simulink model "LCTC_vTD8_Test_TC0_ref6_2007a_TC"  (Simulink 7.0)
settings: Solver=ode4  StartTime=0.0  StopTime=110  FixedStep=0.01  RelTol=1e-3
InitFcn:
  | PedalInterp_para
values from neighbouring files (symbols this model uses):
  ped_min = 5    [PedalInterp_para.m]
  T_max_Brake = 4000    [PedalInterp_para.m]
  TI = 0    [PAS_callback.m]    (also in PSTI_callback.m = [block '/TI'])
undefined symbols (not defined in the file or in its folder): fzgbib, m_ab_100pro, m_ab_70pro, PedalInterpretation, v_bereich
libraries: simulink x320
(part 19 of 50)

== system LCTC_vTD8_Test_TC0_ref6_2007a_TC/TestData 1/SR-PI-02.2 Acceleration pedal interpretation/v = const & phi_Acc increases & T_des_Drive < 0/Generate increase 7/Generate  limited increase for partition1 variant2 1/Subsystem ==
  In1 : Inport  OutDataType=sfix(16)  OutScaling=2^0
  In2 : Inport  Port=2  OutDataType=sfix(16)  OutScaling=2^0
  In3 : Inport  Port=3  OutDataType=sfix(16)  OutScaling=2^0
  Subsystem1 : Subsystem
  Subsystem10 : Subsystem
  Subsystem11 : Subsystem
  Subsystem12 : Subsystem
  Subsystem13 : Subsystem
  Subsystem14 : Subsystem
  Subsystem15 : Subsystem
  Subsystem16 : Subsystem
  Subsystem17 : Subsystem
  Subsystem18 : Subsystem
  Subsystem19 : Subsystem
  Subsystem2 : Subsystem
  Subsystem20 : Subsystem
  Subsystem21 : Subsystem
  Subsystem22 : Subsystem
  Subsystem23 : Subsystem
  Subsystem24 : Subsystem
  Subsystem25 : Subsystem
  Subsystem26 : Subsystem
  Subsystem27 : Subsystem
  Subsystem28 : Subsystem
  Subsystem29 : Subsystem
  Subsystem3 : Subsystem
  Subsystem30 : Subsystem
  Subsystem31 : Subsystem
  Subsystem32 : Subsystem
  Subsystem33 : Subsystem
  Subsystem34 : Subsystem
  Subsystem35 : Subsystem
  Subsystem36 : Subsystem
  Subsystem37 : Subsystem
  Subsystem38 : Subsystem
  Subsystem39 : Subsystem
  Subsystem4 : Subsystem
  Subsystem40 : Subsystem
  Subsystem41 : Subsystem
  Subsystem42 : Subsystem
  Subsystem43 : Subsystem
  Subsystem44 : Subsystem
  Subsystem45 : Subsystem
  Subsystem46 : Subsystem
  Subsystem47 : Subsystem
  Subsystem48 : Subsystem
  Subsystem49 : Subsystem
  Subsystem5 : Subsystem
  Subsystem50 : Subsystem
  Subsystem51 : Subsystem
  Subsystem52 : Subsystem
  Subsystem53 : Subsystem
  Subsystem54 : Subsystem
  Subsystem55 : Subsystem
  Subsystem56 : Subsystem
  Subsystem57 : Subsystem
  Subsystem58 : Subsystem
  Subsystem59 : Subsystem
  Subsystem6 : Subsystem
  Subsystem60 : Subsystem
  Subsystem61 : Subsystem
  Subsystem62 : Subsystem
  Subsystem63 : Subsystem
  Subsystem64 : Subsystem
  Subsystem65 : Subsystem
  Subsystem66 : Subsystem
  Subsystem67 : Subsystem
  Subsystem68 : Subsystem
  Subsystem69 : Subsystem
  Subsystem7 : Subsystem
  Subsystem70 : Subsystem
  Subsystem8 : Subsystem
  Subsystem9 : Subsystem
  Out1 : Outport  OutDataType=sfix(16)  OutScaling=2^0
  wires:
  Subsystem70/1(Out1) -> Out1/1
  Subsystem68/1(Out1) -> Subsystem59/2(In2)
  Subsystem50/1(Out1) -> Subsystem51/2(In2)
  Subsystem69/1(Out1) -> Subsystem70/2(In2)
  Subsystem66/1(Out1) -> Subsystem69/2(In2)
  Subsystem65/1(Out1) -> Subsystem66/2(In2)
  Subsystem64/1(Out1) -> Subsystem65/2(In2)
  Subsystem63/1(Out1) -> Subsystem64/2(In2)
  Subsystem62/1(Out1) -> Subsystem63/2(In2)
  Subsystem61/1(Out1) -> Subsystem62/2(In2)
  Subsystem60/1(Out1) -> Subsystem61/2(In2)
  Subsystem59/1(Out1) -> Subsystem60/2(In2)
  Subsystem67/1(Out1) -> Subsystem68/2(In2)
  Subsystem58/1(Out1) -> Subsystem67/2(In2)
  Subsystem57/1(Out1) -> Subsystem58/2(In2)
  Subsystem56/1(Out1) -> Subsystem57/2(In2)
  Subsystem55/1(Out1) -> Subsystem56/2(In2)
  Subsystem54/1(Out1) -> Subsystem55/2(In2)
  Subsystem53/1(Out1) -> Subsystem54/2(In2)
  Subsystem52/1(Out1) -> Subsystem53/2(In2)
  Subsystem51/1(Out1) -> Subsystem52/2(In2)
  Subsystem48/1(Out1) -> Subsystem35/2(In2)
  Subsystem46/1(Out1) -> Subsystem27/2(In2)
  Subsystem44/1(Out1) -> Subsystem19/2(In2)
  Subsystem49/1(Out1) -> Subsystem50/2(In2)
  Subsystem42/1(Out1) -> Subsystem49/2(In2)
  Subsystem41/1(Out1) -> Subsystem42/2(In2)
  Subsystem40/1(Out1) -> Subsystem41/2(In2)
  Subsystem39/1(Out1) -> Subsystem40/2(In2)
  Subsystem38/1(Out1) -> Subsystem39/2(In2)
  Subsystem37/1(Out1) -> Subsystem38/2(In2)
  Subsystem36/1(Out1) -> Subsystem37/2(In2)
  Subsystem35/1(Out1) -> Subsystem36/2(In2)
  Subsystem47/1(Out1) -> Subsystem48/2(In2)
  Subsystem34/1(Out1) -> Subsystem47/2(In2)
  Subsystem33/1(Out1) -> Subsystem34/2(In2)
  Subsystem32/1(Out1) -> Subsystem33/2(In2)
  Subsystem31/1(Out1) -> Subsystem32/2(In2)
  Subsystem30/1(Out1) -> Subsystem31/2(In2)
  Subsystem29/1(Out1) -> Subsystem30/2(In2)
  Subsystem28/1(Out1) -> Subsystem29/2(In2)
  Subsystem27/1(Out1) -> Subsystem28/2(In2)
  Subsystem45/1(Out1) -> Subsystem46/2(In2)
  Subsystem26/1(Out1) -> Subsystem45/2(In2)
  Subsystem25/1(Out1) -> Subsystem26/2(In2)
  Subsystem23/1(Out1) -> Subsystem24/2(In2)
  Subsystem24/1(Out1) -> Subsystem25/2(In2)
  Subsystem22/1(Out1) -> Subsystem23/2(In2)
  Subsystem21/1(Out1) -> Subsystem22/2(In2)
  Subsystem20/1(Out1) -> Subsystem21/2(In2)
  Subsystem19/1(Out1) -> Subsystem20/2(In2)
  Subsystem43/1(Out1) -> Subsystem44/2(In2)
  Subsystem18/1(Out1) -> Subsystem43/2(In2)
  Subsystem10/1(Out1) -> Subsystem11/2(In2)
  Subsystem17/1(Out1) -> Subsystem18/2(In2)
  Subsystem16/1(Out1) -> Subsystem17/2(In2)
  Subsystem15/1(Out1) -> Subsystem16/2(In2)
  Subsystem13/1(Out1) -> Subsystem14/2(In2)
  Subsystem14/1(Out1) -> Subsystem15/2(In2)
  Subsystem12/1(Out1) -> Subsystem13/2(In2)
  Subsystem11/1(Out1) -> Subsystem12/2(In2)
  Subsystem9/1(Out1) -> Subsystem10/2(In2)
  Subsystem8/1(Out1) -> Subsystem9/2(In2)
  Subsystem7/1(Out1) -> Subsystem8/2(In2)
  Subsystem6/1(Out1) -> Subsystem7/2(In2)
  Subsystem5/1(Out1) -> Subsystem6/2(In2)
  Subsystem4/1(Out1) -> Subsystem5/2(In2)
  Subsystem3/1(Out1) -> Subsystem4/2(In2)
  Subsystem2/1(Out1) -> Subsystem3/2(In2)
  Subsystem1/1(Out1) -> Subsystem2/2(In2)
  In3/1 -> Subsystem70/3(In3), Subsystem69/3(In3), Subsystem66/3(In3), Subsystem65/3(In3), Subsystem64/3(In3), Subsystem63/3(In3), Subsystem62/3(In3), Subsystem61/3(In3), Subsystem60/3(In3), Subsystem59/3(In3), Subsystem68/3(In3), Subsystem67/3(In3), Subsystem58/3(In3), Subsystem57/3(In3), Subsystem56/3(In3), Subsystem55/3(In3), Subsystem54/3(In3), Subsystem53/3(In3), Subsystem52/3(In3), Subsystem51/3(In3), Subsystem50/3(In3), Subsystem49/3(In3), Subsystem42/3(In3), Subsystem41/3(In3), Subsystem40/3(In3), Subsystem39/3(In3), Subsystem38/3(In3), Subsystem37/3(In3), Subsystem36/3(In3), Subsystem35/3(In3), Subsystem48/3(In3), Subsystem47/3(In3), Subsystem34/3(In3), Subsystem33/3(In3), Subsystem32/3(In3), Subsystem31/3(In3), Subsystem30/3(In3), Subsystem29/3(In3), Subsystem28/3(In3), Subsystem27/3(In3), Subsystem46/3(In3), Subsystem45/3(In3), Subsystem26/3(In3), Subsystem25/3(In3), Subsystem24/3(In3), Subsystem23/3(In3), Subsystem22/3(In3), Subsystem21/3(In3), Subsystem20/3(In3), Subsystem19/3(In3), Subsystem11/3(In3), Subsystem44/3(In3), Subsystem43/3(In3), Subsystem18/3(In3), Subsystem17/3(In3), Subsystem16/3(In3), Subsystem15/3(In3), Subsystem14/3(In3), Subsystem13/3(In3), Subsystem12/3(In3), Subsystem1/3(In3), Subsystem10/3(In3), Subsystem9/3(In3), Subsystem8/3(In3), Subsystem7/3(In3), Subsystem6/3(In3), Subsystem5/3(In3), Subsystem4/3(In3), Subsystem3/3(In3), Subsystem2/3(In3)
  In2/1 -> Subsystem1/2(In2)
  In1/1 -> Subsystem44/1(In1), Subsystem43/1(In1), Subsystem18/1(In1), Subsystem17/1(In1), Subsystem16/1(In1), Subsystem15/1(In1), Subsystem14/1(In1), Subsystem13/1(In1), Subsystem12/1(In1), Subsystem11/1(In1), Subsystem46/1(In1), Subsystem45/1(In1), Subsystem26/1(In1), Subsystem25/1(In1), Subsystem24/1(In1), Subsystem23/1(In1), Subsystem22/1(In1), Subsystem21/1(In1), Subsystem20/1(In1), Subsystem19/1(In1), Subsystem70/1(In1), Subsystem69/1(In1), Subsystem66/1(In1), Subsystem65/1(In1), Subsystem64/1(In1), Subsystem63/1(In1), Subsystem62/1(In1), Subsystem61/1(In1), Subsystem60/1(In1), Subsystem59/1(In1), Subsystem68/1(In1), Subsystem67/1(In1), Subsystem58/1(In1), Subsystem57/1(In1), Subsystem56/1(In1), Subsystem55/1(In1), Subsystem54/1(In1), Subsystem53/1(In1), Subsystem52/1(In1), Subsystem51/1(In1), Subsystem50/1(In1), Subsystem49/1(In1), Subsystem42/1(In1), Subsystem41/1(In1), Subsystem40/1(In1), Subsystem39/1(In1), Subsystem38/1(In1), Subsystem37/1(In1), Subsystem36/1(In1), Subsystem35/1(In1), Subsystem48/1(In1), Subsystem47/1(In1), Subsystem34/1(In1), Subsystem33/1(In1), Subsystem32/1(In1), Subsystem31/1(In1), Subsystem30/1(In1), Subsystem29/1(In1), Subsystem28/1(In1), Subsystem27/1(In1), Subsystem10/1(In1), Subsystem9/1(In1), Subsystem8/1(In1), Subsystem7/1(In1), Subsystem6/1(In1), Subsystem5/1(In1), Subsystem4/1(In1), Subsystem3/1(In1), Subsystem2/1(In1), Subsystem1/1(In1)

== system LCTC_vTD8_Test_TC0_ref6_2007a_TC/TestData 1/SR-PI-02.2 Acceleration pedal interpretation/v = const & phi_Acc increases & T_des_Drive >= 0/Generate constant 6/Generate  constant for partition1 variant1 1/Repeating Sequence ==
  Clock : Clock
      settings: Decimation=10
  Fcn1 : Fcn  Expr=rem(u[1],period)
  Look-Up Table1 : Lookup  InputValues=rep_seq_t - min(rep_seq_t)  Table=rep_seq_y  OutDataType=sfix(16)  OutScaling=2^0  SaturateOnIntegerOverflow=on
  Output : SignalConversion  ConversionOutput=Contiguous copy
  Sum : Sum  Inputs=+-  OutDataType=sfix(16)  OutScaling=2^0  SaturateOnIntegerOverflow=on  InputSameDT=on
  startTime : S-Function  FunctionName=sfun_tstart
  Out1 : Outport  OutDataType=sfix(16)  OutScaling=2^0
  wires:
  Output/1 -> Out1/1
  Look-Up Table1/1 -> Output/1
  startTime/1 -> Sum/2
  Clock/1 -> Sum/1
  Sum/1 -> Fcn1/1
  Fcn1/1 -> Look-Up Table1/1

== system LCTC_vTD8_Test_TC0_ref6_2007a_TC/TestData 1/SR-PI-02.2 Acceleration pedal interpretation/v = const & phi_Acc increases & T_des_Drive >= 0/Generate constant 6/Generate  constant for partition1 variant2 1/Repeating Sequence ==
  Clock : Clock
      settings: Decimation=10
  Fcn1 : Fcn  Expr=rem(u[1],period)
  Look-Up Table1 : Lookup  InputValues=rep_seq_t - min(rep_seq_t)  Table=rep_seq_y  OutDataType=sfix(16)  OutScaling=2^0  SaturateOnIntegerOverflow=on
  Output : SignalConversion  ConversionOutput=Contiguous copy
  Sum : Sum  Inputs=+-  OutDataType=sfix(16)  OutScaling=2^0  SaturateOnIntegerOverflow=on  InputSameDT=on
  startTime : S-Function  FunctionName=sfun_tstart
  Out1 : Outport  OutDataType=sfix(16)  OutScaling=2^0
  wires:
  Output/1 -> Out1/1
  Look-Up Table1/1 -> Output/1
  startTime/1 -> Sum/2
  Clock/1 -> Sum/1
  Sum/1 -> Fcn1/1
  Fcn1/1 -> Look-Up Table1/1

== system LCTC_vTD8_Test_TC0_ref6_2007a_TC/TestData 1/SR-PI-02.2 Acceleration pedal interpretation/v = const & phi_Acc increases & T_des_Drive >= 0/Generate constant 6/Generate  constant for partition1 variant3 1/Repeating Sequence ==
  Clock : Clock
      settings: Decimation=10
  Fcn1 : Fcn  Expr=rem(u[1],period)
  Look-Up Table1 : Lookup  InputValues=rep_seq_t - min(rep_seq_t)  Table=rep_seq_y  OutDataType=sfix(16)  OutScaling=2^0  SaturateOnIntegerOverflow=on
  Output : SignalConversion  ConversionOutput=Contiguous copy
  Sum : Sum  Inputs=+-  OutDataType=sfix(16)  OutScaling=2^0  SaturateOnIntegerOverflow=on  InputSameDT=on
  startTime : S-Function  FunctionName=sfun_tstart
  Out1 : Outport  OutDataType=sfix(16)  OutScaling=2^0
  wires:
  Output/1 -> Out1/1
  Look-Up Table1/1 -> Output/1
  startTime/1 -> Sum/2
  Clock/1 -> Sum/1
  Sum/1 -> Fcn1/1
  Fcn1/1 -> Look-Up Table1/1

== system LCTC_vTD8_Test_TC0_ref6_2007a_TC/TestData 1/SR-PI-02.2 Acceleration pedal interpretation/v = const & phi_Acc increases & T_des_Drive >= 0/Generate constant 6/Generate  constant for partition1 variant4 1/Repeating Sequence ==
  Clock : Clock
      settings: Decimation=10
  Fcn1 : Fcn  Expr=rem(u[1],period)
  Look-Up Table1 : Lookup  InputValues=rep_seq_t - min(rep_seq_t)  Table=rep_seq_y  OutDataType=sfix(16)  OutScaling=2^0  SaturateOnIntegerOverflow=on
  Output : SignalConversion  ConversionOutput=Contiguous copy
  Sum : Sum  Inputs=+-  OutDataType=sfix(16)  OutScaling=2^0  SaturateOnIntegerOverflow=on  InputSameDT=on
  startTime : S-Function  FunctionName=sfun_tstart
  Out1 : Outport  OutDataType=sfix(16)  OutScaling=2^0
  wires:
  Output/1 -> Out1/1
  Look-Up Table1/1 -> Output/1
  startTime/1 -> Sum/2
  Clock/1 -> Sum/1
  Sum/1 -> Fcn1/1
  Fcn1/1 -> Look-Up Table1/1

== system LCTC_vTD8_Test_TC0_ref6_2007a_TC/TestData 1/SR-PI-02.2 Acceleration pedal interpretation/v = const & phi_Acc increases & T_des_Drive >= 0/Generate constant 6/Generate  constant for partition1 variant5 1/Repeating Sequence ==
  Clock : Clock
      settings: Decimation=10
  Fcn1 : Fcn  Expr=rem(u[1],period)
  Look-Up Table1 : Lookup  InputValues=rep_seq_t - min(rep_seq_t)  Table=rep_seq_y  OutDataType=sfix(16)  OutScaling=2^0  SaturateOnIntegerOverflow=on
  Output : SignalConversion  ConversionOutput=Contiguous copy
  Sum : Sum  Inputs=+-  OutDataType=sfix(16)  OutScaling=2^0  SaturateOnIntegerOverflow=on  InputSameDT=on
  startTime : S-Function  FunctionName=sfun_tstart
  Out1 : Outport  OutDataType=sfix(16)  OutScaling=2^0
  wires:
  Output/1 -> Out1/1
  Look-Up Table1/1 -> Output/1
  startTime/1 -> Sum/2
  Clock/1 -> Sum/1
  Sum/1 -> Fcn1/1
  Fcn1/1 -> Look-Up Table1/1

== system LCTC_vTD8_Test_TC0_ref6_2007a_TC/TestData 1/SR-PI-02.2 Acceleration pedal interpretation/v = const & phi_Acc increases & T_des_Drive >= 0/Generate increase 7/Generate  limited increase for partition1 variant1 1/Subsystem ==
  In1 : Inport  OutDataType=sfix(16)  OutScaling=2^0
  In2 : Inport  Port=2  OutDataType=sfix(16)  OutScaling=2^0
  In3 : Inport  Port=3  OutDataType=sfix(16)  OutScaling=2^0
  Subsystem1 : Subsystem
  Subsystem10 : Subsystem
  Subsystem11 : Subsystem
  Subsystem12 : Subsystem
  Subsystem13 : Subsystem
  Subsystem14 : Subsystem
  Subsystem15 : Subsystem
  Subsystem16 : Subsystem
  Subsystem17 : Subsystem
  Subsystem18 : Subsystem
  Subsystem19 : Subsystem
  Subsystem2 : Subsystem
  Subsystem20 : Subsystem
  Subsystem21 : Subsystem
  Subsystem22 : Subsystem
  Subsystem23 : Subsystem
  Subsystem24 : Subsystem
  Subsystem25 : Subsystem
  Subsystem26 : Subsystem
  Subsystem27 : Subsystem
  Subsystem28 : Subsystem
  Subsystem29 : Subsystem
  Subsystem3 : Subsystem
  Subsystem30 : Subsystem
  Subsystem31 : Subsystem
  Subsystem32 : Subsystem
  Subsystem33 : Subsystem
  Subsystem34 : Subsystem
  Subsystem35 : Subsystem
  Subsystem36 : Subsystem
  Subsystem37 : Subsystem
  Subsystem38 : Subsystem
  Subsystem39 : Subsystem
  Subsystem4 : Subsystem
  Subsystem40 : Subsystem
  Subsystem41 : Subsystem
  Subsystem42 : Subsystem
  Subsystem43 : Subsystem
  Subsystem44 : Subsystem
  Subsystem45 : Subsystem
  Subsystem46 : Subsystem
  Subsystem47 : Subsystem
  Subsystem48 : Subsystem
  Subsystem49 : Subsystem
  Subsystem5 : Subsystem
  Subsystem50 : Subsystem
  Subsystem51 : Subsystem
  Subsystem52 : Subsystem
  Subsystem53 : Subsystem
  Subsystem54 : Subsystem
  Subsystem55 : Subsystem
  Subsystem56 : Subsystem
  Subsystem57 : Subsystem
  Subsystem58 : Subsystem
  Subsystem59 : Subsystem
  Subsystem6 : Subsystem
  Subsystem60 : Subsystem
  Subsystem61 : Subsystem
  Subsystem62 : Subsystem
  Subsystem63 : Subsystem
  Subsystem64 : Subsystem
  Subsystem65 : Subsystem
  Subsystem66 : Subsystem
  Subsystem67 : Subsystem
  Subsystem68 : Subsystem
  Subsystem69 : Subsystem
  Subsystem7 : Subsystem
  Subsystem70 : Subsystem
  Subsystem8 : Subsystem
  Subsystem9 : Subsystem
  Out1 : Outport  OutDataType=sfix(16)  OutScaling=2^0
  wires:
  Subsystem70/1(Out1) -> Out1/1
  Subsystem68/1(Out1) -> Subsystem59/2(In2)
  Subsystem50/1(Out1) -> Subsystem51/2(In2)
  Subsystem69/1(Out1) -> Subsystem70/2(In2)
  Subsystem66/1(Out1) -> Subsystem69/2(In2)
  Subsystem65/1(Out1) -> Subsystem66/2(In2)
  Subsystem64/1(Out1) -> Subsystem65/2(In2)
  Subsystem63/1(Out1) -> Subsystem64/2(In2)
  Subsystem62/1(Out1) -> Subsystem63/2(In2)
  Subsystem61/1(Out1) -> Subsystem62/2(In2)
  Subsystem60/1(Out1) -> Subsystem61/2(In2)
  Subsystem59/1(Out1) -> Subsystem60/2(In2)
  Subsystem67/1(Out1) -> Subsystem68/2(In2)
  Subsystem58/1(Out1) -> Subsystem67/2(In2)
  Subsystem57/1(Out1) -> Subsystem58/2(In2)
  Subsystem56/1(Out1) -> Subsystem57/2(In2)
  Subsystem55/1(Out1) -> Subsystem56/2(In2)
  Subsystem54/1(Out1) -> Subsystem55/2(In2)
  Subsystem53/1(Out1) -> Subsystem54/2(In2)
  Subsystem52/1(Out1) -> Subsystem53/2(In2)
  Subsystem51/1(Out1) -> Subsystem52/2(In2)
  Subsystem48/1(Out1) -> Subsystem35/2(In2)
  Subsystem46/1(Out1) -> Subsystem27/2(In2)
  Subsystem44/1(Out1) -> Subsystem19/2(In2)
  Subsystem49/1(Out1) -> Subsystem50/2(In2)
  Subsystem42/1(Out1) -> Subsystem49/2(In2)
  Subsystem41/1(Out1) -> Subsystem42/2(In2)
  Subsystem40/1(Out1) -> Subsystem41/2(In2)
  Subsystem39/1(Out1) -> Subsystem40/2(In2)
  Subsystem38/1(Out1) -> Subsystem39/2(In2)
  Subsystem37/1(Out1) -> Subsystem38/2(In2)
  Subsystem36/1(Out1) -> Subsystem37/2(In2)
  Subsystem35/1(Out1) -> Subsystem36/2(In2)
  Subsystem47/1(Out1) -> Subsystem48/2(In2)
  Subsystem34/1(Out1) -> Subsystem47/2(In2)
  Subsystem33/1(Out1) -> Subsystem34/2(In2)
  Subsystem32/1(Out1) -> Subsystem33/2(In2)
  Subsystem31/1(Out1) -> Subsystem32/2(In2)
  Subsystem30/1(Out1) -> Subsystem31/2(In2)
  Subsystem29/1(Out1) -> Subsystem30/2(In2)
  Subsystem28/1(Out1) -> Subsystem29/2(In2)
  Subsystem27/1(Out1) -> Subsystem28/2(In2)
  Subsystem45/1(Out1) -> Subsystem46/2(In2)
  Subsystem26/1(Out1) -> Subsystem45/2(In2)
  Subsystem25/1(Out1) -> Subsystem26/2(In2)
  Subsystem23/1(Out1) -> Subsystem24/2(In2)
  Subsystem24/1(Out1) -> Subsystem25/2(In2)
  Subsystem22/1(Out1) -> Subsystem23/2(In2)
  Subsystem21/1(Out1) -> Subsystem22/2(In2)
  Subsystem20/1(Out1) -> Subsystem21/2(In2)
  Subsystem19/1(Out1) -> Subsystem20/2(In2)
  Subsystem43/1(Out1) -> Subsystem44/2(In2)
  Subsystem18/1(Out1) -> Subsystem43/2(In2)
  Subsystem10/1(Out1) -> Subsystem11/2(In2)
  Subsystem17/1(Out1) -> Subsystem18/2(In2)
  Subsystem16/1(Out1) -> Subsystem17/2(In2)
  Subsystem15/1(Out1) -> Subsystem16/2(In2)
  Subsystem13/1(Out1) -> Subsystem14/2(In2)
  Subsystem14/1(Out1) -> Subsystem15/2(In2)
  Subsystem12/1(Out1) -> Subsystem13/2(In2)
  Subsystem11/1(Out1) -> Subsystem12/2(In2)
  Subsystem9/1(Out1) -> Subsystem10/2(In2)
  Subsystem8/1(Out1) -> Subsystem9/2(In2)
  Subsystem7/1(Out1) -> Subsystem8/2(In2)
  Subsystem6/1(Out1) -> Subsystem7/2(In2)
  Subsystem5/1(Out1) -> Subsystem6/2(In2)
  Subsystem4/1(Out1) -> Subsystem5/2(In2)
  Subsystem3/1(Out1) -> Subsystem4/2(In2)
  Subsystem2/1(Out1) -> Subsystem3/2(In2)
  Subsystem1/1(Out1) -> Subsystem2/2(In2)
  In3/1 -> Subsystem70/3(In3), Subsystem69/3(In3), Subsystem66/3(In3), Subsystem65/3(In3), Subsystem64/3(In3), Subsystem63/3(In3), Subsystem62/3(In3), Subsystem61/3(In3), Subsystem60/3(In3), Subsystem59/3(In3), Subsystem68/3(In3), Subsystem67/3(In3), Subsystem58/3(In3), Subsystem57/3(In3), Subsystem56/3(In3), Subsystem55/3(In3), Subsystem54/3(In3), Subsystem53/3(In3), Subsystem52/3(In3), Subsystem51/3(In3), Subsystem50/3(In3), Subsystem49/3(In3), Subsystem42/3(In3), Subsystem41/3(In3), Subsystem40/3(In3), Subsystem39/3(In3), Subsystem38/3(In3), Subsystem37/3(In3), Subsystem36/3(In3), Subsystem35/3(In3), Subsystem48/3(In3), Subsystem47/3(In3), Subsystem34/3(In3), Subsystem33/3(In3), Subsystem32/3(In3), Subsystem31/3(In3), Subsystem30/3(In3), Subsystem29/3(In3), Subsystem28/3(In3), Subsystem27/3(In3), Subsystem46/3(In3), Subsystem45/3(In3), Subsystem26/3(In3), Subsystem25/3(In3), Subsystem24/3(In3), Subsystem23/3(In3), Subsystem22/3(In3), Subsystem21/3(In3), Subsystem20/3(In3), Subsystem19/3(In3), Subsystem11/3(In3), Subsystem44/3(In3), Subsystem43/3(In3), Subsystem18/3(In3), Subsystem17/3(In3), Subsystem16/3(In3), Subsystem15/3(In3), Subsystem14/3(In3), Subsystem13/3(In3), Subsystem12/3(In3), Subsystem1/3(In3), Subsystem10/3(In3), Subsystem9/3(In3), Subsystem8/3(In3), Subsystem7/3(In3), Subsystem6/3(In3), Subsystem5/3(In3), Subsystem4/3(In3), Subsystem3/3(In3), Subsystem2/3(In3)
  In2/1 -> Subsystem1/2(In2)
  In1/1 -> Subsystem44/1(In1), Subsystem43/1(In1), Subsystem18/1(In1), Subsystem17/1(In1), Subsystem16/1(In1), Subsystem15/1(In1), Subsystem14/1(In1), Subsystem13/1(In1), Subsystem12/1(In1), Subsystem11/1(In1), Subsystem46/1(In1), Subsystem45/1(In1), Subsystem26/1(In1), Subsystem25/1(In1), Subsystem24/1(In1), Subsystem23/1(In1), Subsystem22/1(In1), Subsystem21/1(In1), Subsystem20/1(In1), Subsystem19/1(In1), Subsystem70/1(In1), Subsystem69/1(In1), Subsystem66/1(In1), Subsystem65/1(In1), Subsystem64/1(In1), Subsystem63/1(In1), Subsystem62/1(In1), Subsystem61/1(In1), Subsystem60/1(In1), Subsystem59/1(In1), Subsystem68/1(In1), Subsystem67/1(In1), Subsystem58/1(In1), Subsystem57/1(In1), Subsystem56/1(In1), Subsystem55/1(In1), Subsystem54/1(In1), Subsystem53/1(In1), Subsystem52/1(In1), Subsystem51/1(In1), Subsystem50/1(In1), Subsystem49/1(In1), Subsystem42/1(In1), Subsystem41/1(In1), Subsystem40/1(In1), Subsystem39/1(In1), Subsystem38/1(In1), Subsystem37/1(In1), Subsystem36/1(In1), Subsystem35/1(In1), Subsystem48/1(In1), Subsystem47/1(In1), Subsystem34/1(In1), Subsystem33/1(In1), Subsystem32/1(In1), Subsystem31/1(In1), Subsystem30/1(In1), Subsystem29/1(In1), Subsystem28/1(In1), Subsystem27/1(In1), Subsystem10/1(In1), Subsystem9/1(In1), Subsystem8/1(In1), Subsystem7/1(In1), Subsystem6/1(In1), Subsystem5/1(In1), Subsystem4/1(In1), Subsystem3/1(In1), Subsystem2/1(In1), Subsystem1/1(In1)

== system LCTC_vTD8_Test_TC0_ref6_2007a_TC/TestData 1/SR-PI-02.2 Acceleration pedal interpretation/v = const & phi_Acc increases & T_des_Drive >= 0/Generate increase 7/Generate  limited increase for partition1 variant2 1/Subsystem ==
  In1 : Inport  OutDataType=sfix(16)  OutScaling=2^0
  In2 : Inport  Port=2  OutDataType=sfix(16)  OutScaling=2^0
  In3 : Inport  Port=3  OutDataType=sfix(16)  OutScaling=2^0
  Subsystem1 : Subsystem
  Subsystem10 : Subsystem
  Subsystem11 : Subsystem
  Subsystem12 : Subsystem
  Subsystem13 : Subsystem
  Subsystem14 : Subsystem
  Subsystem15 : Subsystem
  Subsystem16 : Subsystem
  Subsystem17 : Subsystem
  Subsystem18 : Subsystem
  Subsystem19 : Subsystem
  Subsystem2 : Subsystem
  Subsystem20 : Subsystem
  Subsystem21 : Subsystem
  Subsystem22 : Subsystem
  Subsystem23 : Subsystem
  Subsystem24 : Subsystem
  Subsystem25 : Subsystem
  Subsystem26 : Subsystem
  Subsystem27 : Subsystem
  Subsystem28 : Subsystem
  Subsystem29 : Subsystem
  Subsystem3 : Subsystem
  Subsystem30 : Subsystem
  Subsystem31 : Subsystem
  Subsystem32 : Subsystem
  Subsystem33 : Subsystem
  Subsystem34 : Subsystem
  Subsystem35 : Subsystem
  Subsystem36 : Subsystem
  Subsystem37 : Subsystem
  Subsystem38 : Subsystem
  Subsystem39 : Subsystem
  Subsystem4 : Subsystem
  Subsystem40 : Subsystem
  Subsystem41 : Subsystem
  Subsystem42 : Subsystem
  Subsystem43 : Subsystem
  Subsystem44 : Subsystem
  Subsystem45 : Subsystem
  Subsystem46 : Subsystem
  Subsystem47 : Subsystem
  Subsystem48 : Subsystem
  Subsystem49 : Subsystem
  Subsystem5 : Subsystem
  Subsystem50 : Subsystem
  Subsystem51 : Subsystem
  Subsystem52 : Subsystem
  Subsystem53 : Subsystem
  Subsystem54 : Subsystem
  Subsystem55 : Subsystem
  Subsystem56 : Subsystem
  Subsystem57 : Subsystem
  Subsystem58 : Subsystem
  Subsystem59 : Subsystem
  Subsystem6 : Subsystem
  Subsystem60 : Subsystem
  Subsystem61 : Subsystem
  Subsystem62 : Subsystem
  Subsystem63 : Subsystem
  Subsystem64 : Subsystem
  Subsystem65 : Subsystem
  Subsystem66 : Subsystem
  Subsystem67 : Subsystem
  Subsystem68 : Subsystem
  Subsystem69 : Subsystem
  Subsystem7 : Subsystem
  Subsystem70 : Subsystem
  Subsystem8 : Subsystem
  Subsystem9 : Subsystem
  Out1 : Outport  OutDataType=sfix(16)  OutScaling=2^0
  wires:
  Subsystem70/1(Out1) -> Out1/1
  Subsystem68/1(Out1) -> Subsystem59/2(In2)
  Subsystem50/1(Out1) -> Subsystem51/2(In2)
  Subsystem69/1(Out1) -> Subsystem70/2(In2)
  Subsystem66/1(Out1) -> Subsystem69/2(In2)
  Subsystem65/1(Out1) -> Subsystem66/2(In2)
  Subsystem64/1(Out1) -> Subsystem65/2(In2)
  Subsystem63/1(Out1) -> Subsystem64/2(In2)
  Subsystem62/1(Out1) -> Subsystem63/2(In2)
  Subsystem61/1(Out1) -> Subsystem62/2(In2)
  Subsystem60/1(Out1) -> Subsystem61/2(In2)
  Subsystem59/1(Out1) -> Subsystem60/2(In2)
  Subsystem67/1(Out1) -> Subsystem68/2(In2)
  Subsystem58/1(Out1) -> Subsystem67/2(In2)
  Subsystem57/1(Out1) -> Subsystem58/2(In2)
  Subsystem56/1(Out1) -> Subsystem57/2(In2)
  Subsystem55/1(Out1) -> Subsystem56/2(In2)
  Subsystem54/1(Out1) -> Subsystem55/2(In2)
  Subsystem53/1(Out1) -> Subsystem54/2(In2)
  Subsystem52/1(Out1) -> Subsystem53/2(In2)
  Subsystem51/1(Out1) -> Subsystem52/2(In2)
  Subsystem48/1(Out1) -> Subsystem35/2(In2)
  Subsystem46/1(Out1) -> Subsystem27/2(In2)
  Subsystem44/1(Out1) -> Subsystem19/2(In2)
  Subsystem49/1(Out1) -> Subsystem50/2(In2)
  Subsystem42/1(Out1) -> Subsystem49/2(In2)
  Subsystem41/1(Out1) -> Subsystem42/2(In2)
  Subsystem40/1(Out1) -> Subsystem41/2(In2)
  Subsystem39/1(Out1) -> Subsystem40/2(In2)
  Subsystem38/1(Out1) -> Subsystem39/2(In2)
  Subsystem37/1(Out1) -> Subsystem38/2(In2)
  Subsystem36/1(Out1) -> Subsystem37/2(In2)
  Subsystem35/1(Out1) -> Subsystem36/2(In2)
  Subsystem47/1(Out1) -> Subsystem48/2(In2)
  Subsystem34/1(Out1) -> Subsystem47/2(In2)
  Subsystem33/1(Out1) -> Subsystem34/2(In2)
  Subsystem32/1(Out1) -> Subsystem33/2(In2)
  Subsystem31/1(Out1) -> Subsystem32/2(In2)
  Subsystem30/1(Out1) -> Subsystem31/2(In2)
  Subsystem29/1(Out1) -> Subsystem30/2(In2)
  Subsystem28/1(Out1) -> Subsystem29/2(In2)
  Subsystem27/1(Out1) -> Subsystem28/2(In2)
  Subsystem45/1(Out1) -> Subsystem46/2(In2)
  Subsystem26/1(Out1) -> Subsystem45/2(In2)
  Subsystem25/1(Out1) -> Subsystem26/2(In2)
  Subsystem23/1(Out1) -> Subsystem24/2(In2)
  Subsystem24/1(Out1) -> Subsystem25/2(In2)
  Subsystem22/1(Out1) -> Subsystem23/2(In2)
  Subsystem21/1(Out1) -> Subsystem22/2(In2)
  Subsystem20/1(Out1) -> Subsystem21/2(In2)
  Subsystem19/1(Out1) -> Subsystem20/2(In2)
  Subsystem43/1(Out1) -> Subsystem44/2(In2)
  Subsystem18/1(Out1) -> Subsystem43/2(In2)
  Subsystem10/1(Out1) -> Subsystem11/2(In2)
  Subsystem17/1(Out1) -> Subsystem18/2(In2)
  Subsystem16/1(Out1) -> Subsystem17/2(In2)
  Subsystem15/1(Out1) -> Subsystem16/2(In2)
  Subsystem13/1(Out1) -> Subsystem14/2(In2)
  Subsystem14/1(Out1) -> Subsystem15/2(In2)
  Subsystem12/1(Out1) -> Subsystem13/2(In2)
  Subsystem11/1(Out1) -> Subsystem12/2(In2)
  Subsystem9/1(Out1) -> Subsystem10/2(In2)
  Subsystem8/1(Out1) -> Subsystem9/2(In2)
  Subsystem7/1(Out1) -> Subsystem8/2(In2)
  Subsystem6/1(Out1) -> Subsystem7/2(In2)
  Subsystem5/1(Out1) -> Subsystem6/2(In2)
  Subsystem4/1(Out1) -> Subsystem5/2(In2)
  Subsystem3/1(Out1) -> Subsystem4/2(In2)
  Subsystem2/1(Out1) -> Subsystem3/2(In2)
  Subsystem1/1(Out1) -> Subsystem2/2(In2)
  In3/1 -> Subsystem70/3(In3), Subsystem69/3(In3), Subsystem66/3(In3), Subsystem65/3(In3), Subsystem64/3(In3), Subsystem63/3(In3), Subsystem62/3(In3), Subsystem61/3(In3), Subsystem60/3(In3), Subsystem59/3(In3), Subsystem68/3(In3), Subsystem67/3(In3), Subsystem58/3(In3), Subsystem57/3(In3), Subsystem56/3(In3), Subsystem55/3(In3), Subsystem54/3(In3), Subsystem53/3(In3), Subsystem52/3(In3), Subsystem51/3(In3), Subsystem50/3(In3), Subsystem49/3(In3), Subsystem42/3(In3), Subsystem41/3(In3), Subsystem40/3(In3), Subsystem39/3(In3), Subsystem38/3(In3), Subsystem37/3(In3), Subsystem36/3(In3), Subsystem35/3(In3), Subsystem48/3(In3), Subsystem47/3(In3), Subsystem34/3(In3), Subsystem33/3(In3), Subsystem32/3(In3), Subsystem31/3(In3), Subsystem30/3(In3), Subsystem29/3(In3), Subsystem28/3(In3), Subsystem27/3(In3), Subsystem46/3(In3), Subsystem45/3(In3), Subsystem26/3(In3), Subsystem25/3(In3), Subsystem24/3(In3), Subsystem23/3(In3), Subsystem22/3(In3), Subsystem21/3(In3), Subsystem20/3(In3), Subsystem19/3(In3), Subsystem11/3(In3), Subsystem44/3(In3), Subsystem43/3(In3), Subsystem18/3(In3), Subsystem17/3(In3), Subsystem16/3(In3), Subsystem15/3(In3), Subsystem14/3(In3), Subsystem13/3(In3), Subsystem12/3(In3), Subsystem1/3(In3), Subsystem10/3(In3), Subsystem9/3(In3), Subsystem8/3(In3), Subsystem7/3(In3), Subsystem6/3(In3), Subsystem5/3(In3), Subsystem4/3(In3), Subsystem3/3(In3), Subsystem2/3(In3)
  In2/1 -> Subsystem1/2(In2)
  In1/1 -> Subsystem44/1(In1), Subsystem43/1(In1), Subsystem18/1(In1), Subsystem17/1(In1), Subsystem16/1(In1), Subsystem15/1(In1), Subsystem14/1(In1), Subsystem13/1(In1), Subsystem12/1(In1), Subsystem11/1(In1), Subsystem46/1(In1), Subsystem45/1(In1), Subsystem26/1(In1), Subsystem25/1(In1), Subsystem24/1(In1), Subsystem23/1(In1), Subsystem22/1(In1), Subsystem21/1(In1), Subsystem20/1(In1), Subsystem19/1(In1), Subsystem70/1(In1), Subsystem69/1(In1), Subsystem66/1(In1), Subsystem65/1(In1), Subsystem64/1(In1), Subsystem63/1(In1), Subsystem62/1(In1), Subsystem61/1(In1), Subsystem60/1(In1), Subsystem59/1(In1), Subsystem68/1(In1), Subsystem67/1(In1), Subsystem58/1(In1), Subsystem57/1(In1), Subsystem56/1(In1), Subsystem55/1(In1), Subsystem54/1(In1), Subsystem53/1(In1), Subsystem52/1(In1), Subsystem51/1(In1), Subsystem50/1(In1), Subsystem49/1(In1), Subsystem42/1(In1), Subsystem41/1(In1), Subsystem40/1(In1), Subsystem39/1(In1), Subsystem38/1(In1), Subsystem37/1(In1), Subsystem36/1(In1), Subsystem35/1(In1), Subsystem48/1(In1), Subsystem47/1(In1), Subsystem34/1(In1), Subsystem33/1(In1), Subsystem32/1(In1), Subsystem31/1(In1), Subsystem30/1(In1), Subsystem29/1(In1), Subsystem28/1(In1), Subsystem27/1(In1), Subsystem10/1(In1), Subsystem9/1(In1), Subsystem8/1(In1), Subsystem7/1(In1), Subsystem6/1(In1), Subsystem5/1(In1), Subsystem4/1(In1), Subsystem3/1(In1), Subsystem2/1(In1), Subsystem1/1(In1)

== system LCTC_vTD8_Test_TC0_ref6_2007a_TC/TestData 1/SR-PI-02.2 Acceleration pedal interpretation/v increases & phi_Acc = const & T_des_Drive >= 0/Generate constant 6/Generate  constant for partition1 variant1 1/Repeating Sequence ==
  Clock : Clock
      settings: Decimation=10
  Fcn1 : Fcn  Expr=rem(u[1],period)
  Look-Up Table1 : Lookup  InputValues=rep_seq_t - min(rep_seq_t)  Table=rep_seq_y  OutDataType=sfix(16)  OutScaling=2^0  SaturateOnIntegerOverflow=on
  Output : SignalConversion  ConversionOutput=Contiguous copy
  Sum : Sum  Inputs=+-  OutDataType=sfix(16)  OutScaling=2^0  SaturateOnIntegerOverflow=on  InputSameDT=on
  startTime : S-Function  FunctionName=sfun_tstart
  Out1 : Outport  OutDataType=sfix(16)  OutScaling=2^0
  wires:
  Output/1 -> Out1/1
  Look-Up Table1/1 -> Output/1
  startTime/1 -> Sum/2
  Clock/1 -> Sum/1
  Sum/1 -> Fcn1/1
  Fcn1/1 -> Look-Up Table1/1

== system LCTC_vTD8_Test_TC0_ref6_2007a_TC/TestData 1/SR-PI-02.2 Acceleration pedal interpretation/v increases & phi_Acc = const & T_des_Drive >= 0/Generate constant 6/Generate  constant for partition1 variant2 1/Repeating Sequence ==
  Clock : Clock
      settings: Decimation=10
  Fcn1 : Fcn  Expr=rem(u[1],period)
  Look-Up Table1 : Lookup  InputValues=rep_seq_t - min(rep_seq_t)  Table=rep_seq_y  OutDataType=sfix(16)  OutScaling=2^0  SaturateOnIntegerOverflow=on
  Output : SignalConversion  ConversionOutput=Contiguous copy
  Sum : Sum  Inputs=+-  OutDataType=sfix(16)  OutScaling=2^0  SaturateOnIntegerOverflow=on  InputSameDT=on
  startTime : S-Function  FunctionName=sfun_tstart
  Out1 : Outport  OutDataType=sfix(16)  OutScaling=2^0
  wires:
  Output/1 -> Out1/1
  Look-Up Table1/1 -> Output/1
  startTime/1 -> Sum/2
  Clock/1 -> Sum/1
  Sum/1 -> Fcn1/1
  Fcn1/1 -> Look-Up Table1/1

== system LCTC_vTD8_Test_TC0_ref6_2007a_TC/TestData 1/SR-PI-02.2 Acceleration pedal interpretation/v increases & phi_Acc = const & T_des_Drive >= 0/Generate constant 6/Generate  constant for partition1 variant3 1/Repeating Sequence ==
  Clock : Clock
      settings: Decimation=10
  Fcn1 : Fcn  Expr=rem(u[1],period)
  Look-Up Table1 : Lookup  InputValues=rep_seq_t - min(rep_seq_t)  Table=rep_seq_y  OutDataType=sfix(16)  OutScaling=2^0  SaturateOnIntegerOverflow=on
  Output : SignalConversion  ConversionOutput=Contiguous copy
  Sum : Sum  Inputs=+-  OutDataType=sfix(16)  OutScaling=2^0  SaturateOnIntegerOverflow=on  InputSameDT=on
  startTime : S-Function  FunctionName=sfun_tstart
  Out1 : Outport  OutDataType=sfix(16)  OutScaling=2^0
  wires:
  Output/1 -> Out1/1
  Look-Up Table1/1 -> Output/1
  startTime/1 -> Sum/2
  Clock/1 -> Sum/1
  Sum/1 -> Fcn1/1
  Fcn1/1 -> Look-Up Table1/1

== system LCTC_vTD8_Test_TC0_ref6_2007a_TC/TestData 1/SR-PI-02.2 Acceleration pedal interpretation/v increases & phi_Acc = const & T_des_Drive >= 0/Generate increase 7/Generate  limited increase for partition1 variant1 1/Subsystem ==
  In1 : Inport  OutDataType=sfix(16)  OutScaling=2^0
  In2 : Inport  Port=2  OutDataType=sfix(16)  OutScaling=2^0
  In3 : Inport  Port=3  OutDataType=sfix(16)  OutScaling=2^0
  Subsystem1 : Subsystem
  Subsystem10 : Subsystem
  Subsystem11 : Subsystem
  Subsystem12 : Subsystem
  Subsystem13 : Subsystem
  Subsystem14 : Subsystem
  Subsystem15 : Subsystem
  Subsystem16 : Subsystem
  Subsystem17 : Subsystem
  Subsystem18 : Subsystem
  Subsystem19 : Subsystem
  Subsystem2 : Subsystem
  Subsystem20 : Subsystem
  Subsystem21 : Subsystem
  Subsystem22 : Subsystem
  Subsystem23 : Subsystem
  Subsystem24 : Subsystem
  Subsystem25 : Subsystem
  Subsystem26 : Subsystem
  Subsystem27 : Subsystem
  Subsystem28 : Subsystem
  Subsystem29 : Subsystem
  Subsystem3 : Subsystem
  Subsystem30 : Subsystem
  Subsystem31 : Subsystem
  Subsystem32 : Subsystem
  Subsystem33 : Subsystem
  Subsystem34 : Subsystem
  Subsystem35 : Subsystem
  Subsystem36 : Subsystem
  Subsystem37 : Subsystem
  Subsystem38 : Subsystem
  Subsystem39 : Subsystem
  Subsystem4 : Subsystem
  Subsystem40 : Subsystem
  Subsystem41 : Subsystem
  Subsystem42 : Subsystem
  Subsystem43 : Subsystem
  Subsystem44 : Subsystem
  Subsystem45 : Subsystem
  Subsystem46 : Subsystem
  Subsystem47 : Subsystem
  Subsystem48 : Subsystem
  Subsystem49 : Subsystem
  Subsystem5 : Subsystem
  Subsystem50 : Subsystem
  Subsystem51 : Subsystem
  Subsystem52 : Subsystem
  Subsystem53 : Subsystem
  Subsystem54 : Subsystem
  Subsystem55 : Subsystem
  Subsystem56 : Subsystem
  Subsystem57 : Subsystem
  Subsystem58 : Subsystem
  Subsystem59 : Subsystem
  Subsystem6 : Subsystem
  Subsystem60 : Subsystem
  Subsystem61 : Subsystem
  Subsystem62 : Subsystem
  Subsystem63 : Subsystem
  Subsystem64 : Subsystem
  Subsystem65 : Subsystem
  Subsystem66 : Subsystem
  Subsystem67 : Subsystem
  Subsystem68 : Subsystem
  Subsystem69 : Subsystem
  Subsystem7 : Subsystem
  Subsystem70 : Subsystem
  Subsystem8 : Subsystem
  Subsystem9 : Subsystem
  Out1 : Outport  OutDataType=sfix(16)  OutScaling=2^0
  wires:
  Subsystem70/1(Out1) -> Out1/1
  Subsystem68/1(Out1) -> Subsystem59/2(In2)
  Subsystem50/1(Out1) -> Subsystem51/2(In2)
  Subsystem69/1(Out1) -> Subsystem70/2(In2)
  Subsystem66/1(Out1) -> Subsystem69/2(In2)
  Subsystem65/1(Out1) -> Subsystem66/2(In2)
  Subsystem64/1(Out1) -> Subsystem65/2(In2)
  Subsystem63/1(Out1) -> Subsystem64/2(In2)
  Subsystem62/1(Out1) -> Subsystem63/2(In2)
  Subsystem61/1(Out1) -> Subsystem62/2(In2)
  Subsystem60/1(Out1) -> Subsystem61/2(In2)
  Subsystem59/1(Out1) -> Subsystem60/2(In2)
  Subsystem67/1(Out1) -> Subsystem68/2(In2)
  Subsystem58/1(Out1) -> Subsystem67/2(In2)
  Subsystem57/1(Out1) -> Subsystem58/2(In2)
  Subsystem56/1(Out1) -> Subsystem57/2(In2)
  Subsystem55/1(Out1) -> Subsystem56/2(In2)
  Subsystem54/1(Out1) -> Subsystem55/2(In2)
  Subsystem53/1(Out1) -> Subsystem54/2(In2)
  Subsystem52/1(Out1) -> Subsystem53/2(In2)
  Subsystem51/1(Out1) -> Subsystem52/2(In2)
  Subsystem48/1(Out1) -> Subsystem35/2(In2)
  Subsystem46/1(Out1) -> Subsystem27/2(In2)
  Subsystem44/1(Out1) -> Subsystem19/2(In2)
  Subsystem49/1(Out1) -> Subsystem50/2(In2)
  Subsystem42/1(Out1) -> Subsystem49/2(In2)
  Subsystem41/1(Out1) -> Subsystem42/2(In2)
  Subsystem40/1(Out1) -> Subsystem41/2(In2)
  Subsystem39/1(Out1) -> Subsystem40/2(In2)
  Subsystem38/1(Out1) -> Subsystem39/2(In2)
  Subsystem37/1(Out1) -> Subsystem38/2(In2)
  Subsystem36/1(Out1) -> Subsystem37/2(In2)
  Subsystem35/1(Out1) -> Subsystem36/2(In2)
  Subsystem47/1(Out1) -> Subsystem48/2(In2)
  Subsystem34/1(Out1) -> Subsystem47/2(In2)
  Subsystem33/1(Out1) -> Subsystem34/2(In2)
  Subsystem32/1(Out1) -> Subsystem33/2(In2)
  Subsystem31/1(Out1) -> Subsystem32/2(In2)
  Subsystem30/1(Out1) -> Subsystem31/2(In2)
  Subsystem29/1(Out1) -> Subsystem30/2(In2)
  Subsystem28/1(Out1) -> Subsystem29/2(In2)
  Subsystem27/1(Out1) -> Subsystem28/2(In2)
  Subsystem45/1(Out1) -> Subsystem46/2(In2)
  Subsystem26/1(Out1) -> Subsystem45/2(In2)
  Subsystem25/1(Out1) -> Subsystem26/2(In2)
  Subsystem23/1(Out1) -> Subsystem24/2(In2)
  Subsystem24/1(Out1) -> Subsystem25/2(In2)
  Subsystem22/1(Out1) -> Subsystem23/2(In2)
  Subsystem21/1(Out1) -> Subsystem22/2(In2)
  Subsystem20/1(Out1) -> Subsystem21/2(In2)
  Subsystem19/1(Out1) -> Subsystem20/2(In2)
  Subsystem43/1(Out1) -> Subsystem44/2(In2)
  Subsystem18/1(Out1) -> Subsystem43/2(In2)
  Subsystem10/1(Out1) -> Subsystem11/2(In2)
  Subsystem17/1(Out1) -> Subsystem18/2(In2)
  Subsystem16/1(Out1) -> Subsystem17/2(In2)
  Subsystem15/1(Out1) -> Subsystem16/2(In2)
  Subsystem13/1(Out1) -> Subsystem14/2(In2)
  Subsystem14/1(Out1) -> Subsystem15/2(In2)
  Subsystem12/1(Out1) -> Subsystem13/2(In2)
  Subsystem11/1(Out1) -> Subsystem12/2(In2)
  Subsystem9/1(Out1) -> Subsystem10/2(In2)
  Subsystem8/1(Out1) -> Subsystem9/2(In2)
  Subsystem7/1(Out1) -> Subsystem8/2(In2)
  Subsystem6/1(Out1) -> Subsystem7/2(In2)
  Subsystem5/1(Out1) -> Subsystem6/2(In2)
  Subsystem4/1(Out1) -> Subsystem5/2(In2)
  Subsystem3/1(Out1) -> Subsystem4/2(In2)
  Subsystem2/1(Out1) -> Subsystem3/2(In2)
  Subsystem1/1(Out1) -> Subsystem2/2(In2)
  In3/1 -> Subsystem70/3(In3), Subsystem69/3(In3), Subsystem66/3(In3), Subsystem65/3(In3), Subsystem64/3(In3), Subsystem63/3(In3), Subsystem62/3(In3), Subsystem61/3(In3), Subsystem60/3(In3), Subsystem59/3(In3), Subsystem68/3(In3), Subsystem67/3(In3), Subsystem58/3(In3), Subsystem57/3(In3), Subsystem56/3(In3), Subsystem55/3(In3), Subsystem54/3(In3), Subsystem53/3(In3), Subsystem52/3(In3), Subsystem51/3(In3), Subsystem50/3(In3), Subsystem49/3(In3), Subsystem42/3(In3), Subsystem41/3(In3), Subsystem40/3(In3), Subsystem39/3(In3), Subsystem38/3(In3), Subsystem37/3(In3), Subsystem36/3(In3), Subsystem35/3(In3), Subsystem48/3(In3), Subsystem47/3(In3), Subsystem34/3(In3), Subsystem33/3(In3), Subsystem32/3(In3), Subsystem31/3(In3), Subsystem30/3(In3), Subsystem29/3(In3), Subsystem28/3(In3), Subsystem27/3(In3), Subsystem46/3(In3), Subsystem45/3(In3), Subsystem26/3(In3), Subsystem25/3(In3), Subsystem24/3(In3), Subsystem23/3(In3), Subsystem22/3(In3), Subsystem21/3(In3), Subsystem20/3(In3), Subsystem19/3(In3), Subsystem11/3(In3), Subsystem44/3(In3), Subsystem43/3(In3), Subsystem18/3(In3), Subsystem17/3(In3), Subsystem16/3(In3), Subsystem15/3(In3), Subsystem14/3(In3), Subsystem13/3(In3), Subsystem12/3(In3), Subsystem1/3(In3), Subsystem10/3(In3), Subsystem9/3(In3), Subsystem8/3(In3), Subsystem7/3(In3), Subsystem6/3(In3), Subsystem5/3(In3), Subsystem4/3(In3), Subsystem3/3(In3), Subsystem2/3(In3)
  In2/1 -> Subsystem1/2(In2)
  In1/1 -> Subsystem44/1(In1), Subsystem43/1(In1), Subsystem18/1(In1), Subsystem17/1(In1), Subsystem16/1(In1), Subsystem15/1(In1), Subsystem14/1(In1), Subsystem13/1(In1), Subsystem12/1(In1), Subsystem11/1(In1), Subsystem46/1(In1), Subsystem45/1(In1), Subsystem26/1(In1), Subsystem25/1(In1), Subsystem24/1(In1), Subsystem23/1(In1), Subsystem22/1(In1), Subsystem21/1(In1), Subsystem20/1(In1), Subsystem19/1(In1), Subsystem70/1(In1), Subsystem69/1(In1), Subsystem66/1(In1), Subsystem65/1(In1), Subsystem64/1(In1), Subsystem63/1(In1), Subsystem62/1(In1), Subsystem61/1(In1), Subsystem60/1(In1), Subsystem59/1(In1), Subsystem68/1(In1), Subsystem67/1(In1), Subsystem58/1(In1), Subsystem57/1(In1), Subsystem56/1(In1), Subsystem55/1(In1), Subsystem54/1(In1), Subsystem53/1(In1), Subsystem52/1(In1), Subsystem51/1(In1), Subsystem50/1(In1), Subsystem49/1(In1), Subsystem42/1(In1), Subsystem41/1(In1), Subsystem40/1(In1), Subsystem39/1(In1), Subsystem38/1(In1), Subsystem37/1(In1), Subsystem36/1(In1), Subsystem35/1(In1), Subsystem48/1(In1), Subsystem47/1(In1), Subsystem34/1(In1), Subsystem33/1(In1), Subsystem32/1(In1), Subsystem31/1(In1), Subsystem30/1(In1), Subsystem29/1(In1), Subsystem28/1(In1), Subsystem27/1(In1), Subsystem10/1(In1), Subsystem9/1(In1), Subsystem8/1(In1), Subsystem7/1(In1), Subsystem6/1(In1), Subsystem5/1(In1), Subsystem4/1(In1), Subsystem3/1(In1), Subsystem2/1(In1), Subsystem1/1(In1)

== system LCTC_vTD8_Test_TC0_ref6_2007a_TC/TestData 1/SR-PI-02.2 Acceleration pedal interpretation/v increases & phi_Acc = const & T_des_Drive >= 0/Generate increase 7/Generate  limited increase for partition1 variant2 1/Subsystem ==
  In1 : Inport  OutDataType=sfix(16)  OutScaling=2^0
  In2 : Inport  Port=2  OutDataType=sfix(16)  OutScaling=2^0
  In3 : Inport  Port=3  OutDataType=sfix(16)  OutScaling=2^0
  Subsystem1 : Subsystem
  Subsystem10 : Subsystem
  Subsystem11 : Subsystem
  Subsystem12 : Subsystem
  Subsystem13 : Subsystem
  Subsystem14 : Subsystem
  Subsystem15 : Subsystem
  Subsystem16 : Subsystem
  Subsystem17 : Subsystem
  Subsystem18 : Subsystem
  Subsystem19 : Subsystem
  Subsystem2 : Subsystem
  Subsystem20 : Subsystem
  Subsystem21 : Subsystem
  Subsystem22 : Subsystem
  Subsystem23 : Subsystem
  Subsystem24 : Subsystem
  Subsystem25 : Subsystem
  Subsystem26 : Subsystem
  Subsystem27 : Subsystem
  Subsystem28 : Subsystem
  Subsystem29 : Subsystem
  Subsystem3 : Subsystem
  Subsystem30 : Subsystem
  Subsystem31 : Subsystem
  Subsystem32 : Subsystem
  Subsystem33 : Subsystem
  Subsystem34 : Subsystem
  Subsystem35 : Subsystem
  Subsystem36 : Subsystem
  Subsystem37 : Subsystem
  Subsystem38 : Subsystem
  Subsystem39 : Subsystem
  Subsystem4 : Subsystem
  Subsystem40 : Subsystem
  Subsystem41 : Subsystem
  Subsystem42 : Subsystem
  Subsystem43 : Subsystem
  Subsystem44 : Subsystem
  Subsystem45 : Subsystem
  Subsystem46 : Subsystem
  Subsystem47 : Subsystem
  Subsystem48 : Subsystem
  Subsystem49 : Subsystem
  Subsystem5 : Subsystem
  Subsystem50 : Subsystem
  Subsystem51 : Subsystem
  Subsystem52 : Subsystem
  Subsystem53 : Subsystem
  Subsystem54 : Subsystem
  Subsystem55 : Subsystem
  Subsystem56 : Subsystem
  Subsystem57 : Subsystem
  Subsystem58 : Subsystem
  Subsystem59 : Subsystem
  Subsystem6 : Subsystem
  Subsystem60 : Subsystem
  Subsystem61 : Subsystem
  Subsystem62 : Subsystem
  Subsystem63 : Subsystem
  Subsystem64 : Subsystem
  Subsystem65 : Subsystem
  Subsystem66 : Subsystem
  Subsystem67 : Subsystem
  Subsystem68 : Subsystem
  Subsystem69 : Subsystem
  Subsystem7 : Subsystem
  Subsystem70 : Subsystem
  Subsystem8 : Subsystem
  Subsystem9 : Subsystem
  Out1 : Outport  OutDataType=sfix(16)  OutScaling=2^0
  wires:
  Subsystem70/1(Out1) -> Out1/1
  Subsystem68/1(Out1) -> Subsystem59/2(In2)
  Subsystem50/1(Out1) -> Subsystem51/2(In2)
  Subsystem69/1(Out1) -> Subsystem70/2(In2)
  Subsystem66/1(Out1) -> Subsystem69/2(In2)
  Subsystem65/1(Out1) -> Subsystem66/2(In2)
  Subsystem64/1(Out1) -> Subsystem65/2(In2)
  Subsystem63/1(Out1) -> Subsystem64/2(In2)
  Subsystem62/1(Out1) -> Subsystem63/2(In2)
  Subsystem61/1(Out1) -> Subsystem62/2(In2)
  Subsystem60/1(Out1) -> Subsystem61/2(In2)
  Subsystem59/1(Out1) -> Subsystem60/2(In2)
  Subsystem67/1(Out1) -> Subsystem68/2(In2)
  Subsystem58/1(Out1) -> Subsystem67/2(In2)
  Subsystem57/1(Out1) -> Subsystem58/2(In2)
  Subsystem56/1(Out1) -> Subsystem57/2(In2)
  Subsystem55/1(Out1) -> Subsystem56/2(In2)
  Subsystem54/1(Out1) -> Subsystem55/2(In2)
  Subsystem53/1(Out1) -> Subsystem54/2(In2)
  Subsystem52/1(Out1) -> Subsystem53/2(In2)
  Subsystem51/1(Out1) -> Subsystem52/2(In2)
  Subsystem48/1(Out1) -> Subsystem35/2(In2)
  Subsystem46/1(Out1) -> Subsystem27/2(In2)
  Subsystem44/1(Out1) -> Subsystem19/2(In2)
  Subsystem49/1(Out1) -> Subsystem50/2(In2)
  Subsystem42/1(Out1) -> Subsystem49/2(In2)
  Subsystem41/1(Out1) -> Subsystem42/2(In2)
  Subsystem40/1(Out1) -> Subsystem41/2(In2)
  Subsystem39/1(Out1) -> Subsystem40/2(In2)
  Subsystem38/1(Out1) -> Subsystem39/2(In2)
  Subsystem37/1(Out1) -> Subsystem38/2(In2)
  Subsystem36/1(Out1) -> Subsystem37/2(In2)
  Subsystem35/1(Out1) -> Subsystem36/2(In2)
  Subsystem47/1(Out1) -> Subsystem48/2(In2)
  Subsystem34/1(Out1) -> Subsystem47/2(In2)
  Subsystem33/1(Out1) -> Subsystem34/2(In2)
  Subsystem32/1(Out1) -> Subsystem33/2(In2)
  Subsystem31/1(Out1) -> Subsystem32/2(In2)
  Subsystem30/1(Out1) -> Subsystem31/2(In2)
  Subsystem29/1(Out1) -> Subsystem30/2(In2)
  Subsystem28/1(Out1) -> Subsystem29/2(In2)
  Subsystem27/1(Out1) -> Subsystem28/2(In2)
  Subsystem45/1(Out1) -> Subsystem46/2(In2)
  Subsystem26/1(Out1) -> Subsystem45/2(In2)
  Subsystem25/1(Out1) -> Subsystem26/2(In2)
  Subsystem23/1(Out1) -> Subsystem24/2(In2)
  Subsystem24/1(Out1) -> Subsystem25/2(In2)
  Subsystem22/1(Out1) -> Subsystem23/2(In2)
  Subsystem21/1(Out1) -> Subsystem22/2(In2)
  Subsystem20/1(Out1) -> Subsystem21/2(In2)
  Subsystem19/1(Out1) -> Subsystem20/2(In2)
  Subsystem43/1(Out1) -> Subsystem44/2(In2)
  Subsystem18/1(Out1) -> Subsystem43/2(In2)
  Subsystem10/1(Out1) -> Subsystem11/2(In2)
  Subsystem17/1(Out1) -> Subsystem18/2(In2)
  Subsystem16/1(Out1) -> Subsystem17/2(In2)
  Subsystem15/1(Out1) -> Subsystem16/2(In2)
  Subsystem13/1(Out1) -> Subsystem14/2(In2)
  Subsystem14/1(Out1) -> Subsystem15/2(In2)
  Subsystem12/1(Out1) -> Subsystem13/2(In2)
  Subsystem11/1(Out1) -> Subsystem12/2(In2)
  Subsystem9/1(Out1) -> Subsystem10/2(In2)
  Subsystem8/1(Out1) -> Subsystem9/2(In2)
  Subsystem7/1(Out1) -> Subsystem8/2(In2)
  Subsystem6/1(Out1) -> Subsystem7/2(In2)
  Subsystem5/1(Out1) -> Subsystem6/2(In2)
  Subsystem4/1(Out1) -> Subsystem5/2(In2)
  Subsystem3/1(Out1) -> Subsystem4/2(In2)
  Subsystem2/1(Out1) -> Subsystem3/2(In2)
  Subsystem1/1(Out1) -> Subsystem2/2(In2)
  In3/1 -> Subsystem70/3(In3), Subsystem69/3(In3), Subsystem66/3(In3), Subsystem65/3(In3), Subsystem64/3(In3), Subsystem63/3(In3), Subsystem62/3(In3), Subsystem61/3(In3), Subsystem60/3(In3), Subsystem59/3(In3), Subsystem68/3(In3), Subsystem67/3(In3), Subsystem58/3(In3), Subsystem57/3(In3), Subsystem56/3(In3), Subsystem55/3(In3), Subsystem54/3(In3), Subsystem53/3(In3), Subsystem52/3(In3), Subsystem51/3(In3), Subsystem50/3(In3), Subsystem49/3(In3), Subsystem42/3(In3), Subsystem41/3(In3), Subsystem40/3(In3), Subsystem39/3(In3), Subsystem38/3(In3), Subsystem37/3(In3), Subsystem36/3(In3), Subsystem35/3(In3), Subsystem48/3(In3), Subsystem47/3(In3), Subsystem34/3(In3), Subsystem33/3(In3), Subsystem32/3(In3), Subsystem31/3(In3), Subsystem30/3(In3), Subsystem29/3(In3), Subsystem28/3(In3), Subsystem27/3(In3), Subsystem46/3(In3), Subsystem45/3(In3), Subsystem26/3(In3), Subsystem25/3(In3), Subsystem24/3(In3), Subsystem23/3(In3), Subsystem22/3(In3), Subsystem21/3(In3), Subsystem20/3(In3), Subsystem19/3(In3), Subsystem11/3(In3), Subsystem44/3(In3), Subsystem43/3(In3), Subsystem18/3(In3), Subsystem17/3(In3), Subsystem16/3(In3), Subsystem15/3(In3), Subsystem14/3(In3), Subsystem13/3(In3), Subsystem12/3(In3), Subsystem1/3(In3), Subsystem10/3(In3), Subsystem9/3(In3), Subsystem8/3(In3), Subsystem7/3(In3), Subsystem6/3(In3), Subsystem5/3(In3), Subsystem4/3(In3), Subsystem3/3(In3), Subsystem2/3(In3)
  In2/1 -> Subsystem1/2(In2)
  In1/1 -> Subsystem44/1(In1), Subsystem43/1(In1), Subsystem18/1(In1), Subsystem17/1(In1), Subsystem16/1(In1), Subsystem15/1(In1), Subsystem14/1(In1), Subsystem13/1(In1), Subsystem12/1(In1), Subsystem11/1(In1), Subsystem46/1(In1), Subsystem45/1(In1), Subsystem26/1(In1), Subsystem25/1(In1), Subsystem24/1(In1), Subsystem23/1(In1), Subsystem22/1(In1), Subsystem21/1(In1), Subsystem20/1(In1), Subsystem19/1(In1), Subsystem70/1(In1), Subsystem69/1(In1), Subsystem66/1(In1), Subsystem65/1(In1), Subsystem64/1(In1), Subsystem63/1(In1), Subsystem62/1(In1), Subsystem61/1(In1), Subsystem60/1(In1), Subsystem59/1(In1), Subsystem68/1(In1), Subsystem67/1(In1), Subsystem58/1(In1), Subsystem57/1(In1), Subsystem56/1(In1), Subsystem55/1(In1), Subsystem54/1(In1), Subsystem53/1(In1), Subsystem52/1(In1), Subsystem51/1(In1), Subsystem50/1(In1), Subsystem49/1(In1), Subsystem42/1(In1), Subsystem41/1(In1), Subsystem40/1(In1), Subsystem39/1(In1), Subsystem38/1(In1), Subsystem37/1(In1), Subsystem36/1(In1), Subsystem35/1(In1), Subsystem48/1(In1), Subsystem47/1(In1), Subsystem34/1(In1), Subsystem33/1(In1), Subsystem32/1(In1), Subsystem31/1(In1), Subsystem30/1(In1), Subsystem29/1(In1), Subsystem28/1(In1), Subsystem27/1(In1), Subsystem10/1(In1), Subsystem9/1(In1), Subsystem8/1(In1), Subsystem7/1(In1), Subsystem6/1(In1), Subsystem5/1(In1), Subsystem4/1(In1), Subsystem3/1(In1), Subsystem2/1(In1), Subsystem1/1(In1)
